simulink model "fft_16k_core"  (Simulink 7.4)
settings: Solver=ode45  StartTime=0.0  StopTime=10.0  RelTol=1e-3
libraries: xbsIndex_r4 x2252, casper_library_misc x100, casper_library_delays x17, casper_library_ffts_twiddle x1
(part 10 of 41)

== system fft_16k_core/fft_wideband_real/fft_direct/butterfly1_1/twiddle_general_4mult ==
  a : Inport
  b : Inport  Port=2
  sync : Inport  Port=3
  AddSub : xbsIndex_r4/AddSub  mode=Subtraction  use_carryin=off  use_carryout=off  en=off  latency=add_latency  precision=Full  arith_type=Unsigned  n_bits=16  bin_pt=14  quantization=Truncate  overflow=Wrap  dbl_ovrd=off  use_behavioral_HDL=on  hw_selection=Fabric  pipelined=off  block_type=addsub
  AddSub1 : xbsIndex_r4/AddSub  mode=Addition  use_carryin=off  use_carryout=off  en=off  latency=add_latency  precision=Full  arith_type=Unsigned  n_bits=16  bin_pt=14  quantization=Truncate  overflow=Wrap  dbl_ovrd=off  use_behavioral_HDL=on  hw_selection=Fabric  pipelined=off  block_type=addsub
  c_to_ri1 : casper_library_misc/c_to_ri  SystemSampleTime=-1  n_bits=input_bit_width  bin_pt=input_bit_width-1
  c_to_ri2 : casper_library_misc/c_to_ri  SystemSampleTime=-1  n_bits=coeff_bit_width  bin_pt=coeff_bit_width-2
      settings: AttributesFormatString=18_16 r/i
  c_to_ri3 : casper_library_misc/c_to_ri  SystemSampleTime=-1  n_bits=input_bit_width  bin_pt=input_bit_width-1
  coeff_gen : Subsystem  AncestorBlock=casper_library_ffts_twiddle_coeff_gen/coeff_gen  mask(coeff_gen){Coeffs=[1+0i       0.99999970586288222662574298738036-0.0007669903187427044854995727973801i       0.99999882345170187925020854891045-0.0015339801862847655001403923691328i        0.99999735276697820918201387030422-0.002300969151425804985755529941116i       0.99999529380957619117964441102231-0.0030679567629659761432425746363606i       0.99999264658070718958526867936598-0.0038349425697062275442128509439499i       0.99998941108192840321322591989883-0.0046019261204485704952471536444136i       0.99998558731514319841693350099376-0.0053689069639963424990236617873052i       0.99998117528260110908888691483298-0.0061358846491544752690949771078976i       0.99997617498689761461605485237669-0.0069028587247297558057712585366517i        0.99997058643097413987987920336309-0.007669828739531097012982474581122i       0.99996440961811827730087998133968-0.0084367942423697987741615378354254i       0.99995764455196389786095778617891-0.0092037547820598194364682953505508i         0.9999502912364904849695790289843-0.009970709907418030815140497225002i        0.99994234967602391161989316969994-0.010737659167264490545079524963512i        0.99993381987523599629952286704793-0.011504602110422713565074381847353i        0.99992470183914450299056397852837-0.012271538285719925387429185548172i        0.99991499557311347423649294796633-0.013038467241987334449548896486704i        0.99990470108285289807525941796484-0.013805388528060390587737238377031i        0.99989381837441848599468130487367-0.014572301692779064327676330492523i        0.99988234745421256111086449891445-0.015339206284988100154054180279672i        0.99987028832898294794517823902424-0.016106101853537287127426935740004i        0.99985764100582386060267481298069-0.016872987947281710419122902067102i        0.99984440549217523663827478230814-0.017639864115082053153127361611041i        0.99983058179582340319058175737155-0.018406729905804820185410974886508i        0.99981616992490041084806762228254-0.019173584868322622598579840769162i         0.99980116988788425569367745993077-0.01994042855151444137984384497031i        0.99978558169359921237173693953082-0.020707260504265894568431960465205i        0.99976940535121527897644000404398-0.021474080275469507872454499874948i        0.99975264087024884318566364527214-0.022240887414024960999636704173099i        0.99973528826056168306024574121693-0.023007681468839368682521850928424i        0.99971734753236218828931214375189-0.023774461988827554764780458640416i        0.99969881869620424996725205346593-0.024541228522912288123603019585062i        0.99967970176298792672753279475728-0.025307980620024570633797011964816i        0.99965999674395922269809489080217-0.026074717829103900845755248383284i        0.99963970365071019852365452607046-0.026841439699098530724530320412669i        0.99961882249517863829879615877871-0.027608145778965739736143802929291i        0.99959735328964838263487990843714-0.028374835617672098525554602588272i        0.99957529604674921763773909333395-0.029141508764193722186286450437365i        0.99955265077945698592998269305099-0.029908164767516554755077962113319i        0.99952941750109314256178549840115-0.030674803176636625950957082409332i        0.99950559622532531012240042400663-0.031441423540560300975421625935269i        0.99948118696616694567325112075196-0.032208025408304585823771049035713i        0.99945618973797734074793197578401-0.032974608328897335451923567006816i         0.99943060455546173237451057502767-0.03374117185137757990442963773603i         0.9994044314336713030755277031858-0.034507715524795749828523838687033i         0.9993776703880028478010899561923-0.035274238898213947090987119281635i         0.99935032143419944006268451630604-0.03604074152070622927279686109614i        0.99932238458834954375475945198559-0.036807222941358831713731802892653i        0.99929385986688779031084095549886-0.037573682709270493640385524258818i        0.99926474728659442359202103034477-0.038340120373552694088559178453579i ...(+319548 chars)], coeff_bit_width=coeff_bit_width, StepPeriod=0, bram_latency=bram_latency, coeffs_bram=on}
      mask prompts: Coeffs "Coefficients: (0 to 2^(FFTSize-1)-1)"; coeff_bit_width "Coefficient Bit Width:"; StepPeriod "Step Period: (2^?)"; bram_latency "Latency:"; coeffs_bram "Store coefficients in BRAM"
      mask help: Generates a sequence of complex coefficients
      mask init:
      | coeff_gen_init( gcb, ...
      |     'Coeffs', Coeffs, ...
      |     'coeff_bit_width', coeff_bit_width, ...
      |     'StepPeriod', StepPeriod, ...
      |     'bram_latency', bram_latency, ...
      |     'coeffs_bram', coeffs_bram);
      settings: AttributesFormatString=4096 @ (18,16)
      mask CallbackString:
      | Coeffs = eval(get_param(gcb, 'Coeffs'));
      | if( length(Coeffs) == 1 ),
      |     set_param(gcb, 'MaskVisibilities',{'on','on','off','off','off'});
      | else
      |     set_param(gcb, 'MaskVisibilities',{'on','on','on','on','on'});
      | end||||
  convert0 : xbsIndex_r4/Convert  arith_type="Signed  (2's comp)"  n_bits=input_bit_width+4  bin_pt=input_bit_width+1  quantization="Round  (unbiased: +/- Inf)"  overflow=Wrap  latency=conv_latency  dbl_ovrd=off  pipeline=on  block_type=convert
      settings: block_version=11.4
  convert1 : xbsIndex_r4/Convert  arith_type="Signed  (2's comp)"  n_bits=input_bit_width+4  bin_pt=input_bit_width+1  quantization="Round  (unbiased: +/- Inf)"  overflow=Wrap  latency=conv_latency  dbl_ovrd=off  pipeline=on  block_type=convert
      settings: block_version=11.4
  delay0 : xbsIndex_r4/Delay  en=off  latency=mult_latency + add_latency + bram_latency + conv_latency  dbl_ovrd=off  reg_retiming=off  block_type=delay
      settings: block_version=11.4
  delay1 : xbsIndex_r4/Delay  en=off  latency=bram_latency  dbl_ovrd=off  reg_retiming=on  block_type=delay
      settings: block_version=11.4
  delay2 : xbsIndex_r4/Delay  en=off  latency=mult_latency + add_latency + bram_latency + conv_latency  dbl_ovrd=off  reg_retiming=off  block_type=delay
      settings: block_version=11.4
  mult{,1..3} (4 alike) : xbsIndex_r4/Mult  precision=Full  arith_type="Signed  (2's comp)"  n_bits=16  bin_pt=14  quantization=Truncate  overflow=Wrap  en=off  latency=mult_latency  dbl_ovrd=off  use_behavioral_HDL=on  opt=Speed  use_embedded=off  optimum_pipeline=off  pipeline=on  use_rpm=on  placement_style=Triangular  block_type=mult
      settings: block_version=11.4
  a_re : Outport
  a_im : Outport  Port=2
  bw_re : Outport  Port=3
  bw_im : Outport  Port=4
  sync_out : Outport  Port=5
  wires:
  a/1 -> delay0/1
  delay0/1 -> c_to_ri1/1
  c_to_ri1/1 -> a_re/1
  c_to_ri1/2 -> a_im/1
  sync/1 -> delay2/1, coeff_gen/1(rst)
  delay2/1 -> sync_out/1
  coeff_gen/1(w) -> c_to_ri2/1
  b/1 -> delay1/1
  delay1/1 -> c_to_ri3/1
  c_to_ri3/1 -> mult/1, mult3/1
  c_to_ri2/1 -> mult/2, mult1/2
  c_to_ri3/2 -> mult1/1, mult2/1
  c_to_ri2/2 -> mult2/2, mult3/2
  mult/1 -> AddSub/1
  mult1/1 -> AddSub1/1
  mult2/1 -> AddSub/2
  mult3/1 -> AddSub1/2
  AddSub/1 -> convert0/1
  convert0/1 -> bw_re/1
  AddSub1/1 -> convert1/1
  convert1/1 -> bw_im/1
